simulink model "VirtualADAPT"  (Simulink 8.9) (continued)
== system VirtualADAPT/VirtualADAPTv1/PowerDistribution/SensorIT281 ==
  TrueValue : Inport
  MSe : Subsystem  mask{FP=Nominal, FT=0, FM=0}
      mask prompts: FP "Fault Profile:"; FT "Fault Injection Time:"; FM "Fault Magnitude/Slope/Bias/StuckAtValue"
      mask TypeOptions FP: Nominal | Abrupt | Incipient | Bias | StuckAt
      mask Display: image(imread('MSe.png','BackgroundColor',[1 1 1]))
  R : Subsystem  mask{R=1, FP=Nominal, FT=0, FM=0}
      mask prompts: R "Value of R:"; FP "Fault Profile:"; FT "Fault Injection Time:"; FM "Fault Magnitude/Slope"
      mask TypeOptions FP: Nominal | Abrupt | Incipient
      mask Display: image(imread('R.png','BackgroundColor',[1 1 1]))
  TrueValueMod : Subsystem
      mask Display: image(imread('fx.png','BackgroundColor',[1 1 1]))
  Value : Subsystem
      mask Display: image(imread('0.png','BackgroundColor',[1 1 1]))
  SensedValue : Outport
  wires:
  Value/1(y(1)) -> R/1(In)
  R/1(Out) -> Value/1(u(1))
  MSe/1(E) -> Value/2(u(2))
  Value/2(y(2)) -> MSe/1(F)
  TrueValueMod/1(y(1)) -> MSe/2(Se)
  TrueValue/1 -> TrueValueMod/1(u(1))
  Value/3(O) -> SensedValue/1

== system VirtualADAPT/VirtualADAPTv1/PowerDistribution/SensorST165 ==
  TrueValue : Inport
  MSe : Subsystem  mask{FP=Nominal, FT=0, FM=0}
      mask prompts: FP "Fault Profile:"; FT "Fault Injection Time:"; FM "Fault Magnitude/Slope/Bias/StuckAtValue"
      mask TypeOptions FP: Nominal | Abrupt | Incipient | Bias | StuckAt
      mask Display: image(imread('MSe.png','BackgroundColor',[1 1 1]))
  R : Subsystem  mask{R=1, FP=Nominal, FT=0, FM=0}
      mask prompts: R "Value of R:"; FP "Fault Profile:"; FT "Fault Injection Time:"; FM "Fault Magnitude/Slope"
      mask TypeOptions FP: Nominal | Abrupt | Incipient
      mask Display: image(imread('R.png','BackgroundColor',[1 1 1]))
  TrueValueMod : Subsystem
      mask Display: image(imread('fx.png','BackgroundColor',[1 1 1]))
  Value : Subsystem
      mask Display: image(imread('0.png','BackgroundColor',[1 1 1]))
  SensedValue : Outport
  wires:
  Value/1(y(1)) -> R/1(In)
  R/1(Out) -> Value/1(u(1))
  MSe/1(E) -> Value/2(u(2))
  Value/2(y(2)) -> MSe/1(F)
  TrueValueMod/1(y(1)) -> MSe/2(Se)
  TrueValue/1 -> TrueValueMod/1(u(1))
  Value/3(O) -> SensedValue/1

== system VirtualADAPT/VirtualADAPTv1/PowerDistribution/SensorST265 ==
  TrueValue : Inport
  MSe : Subsystem  mask{FP=Nominal, FT=0, FM=0}
      mask prompts: FP "Fault Profile:"; FT "Fault Injection Time:"; FM "Fault Magnitude/Slope/Bias/StuckAtValue"
      mask TypeOptions FP: Nominal | Abrupt | Incipient | Bias | StuckAt
      mask Display: image(imread('MSe.png','BackgroundColor',[1 1 1]))
  R : Subsystem  mask{R=1, FP=Nominal, FT=0, FM=0}
      mask prompts: R "Value of R:"; FP "Fault Profile:"; FT "Fault Injection Time:"; FM "Fault Magnitude/Slope"
      mask TypeOptions FP: Nominal | Abrupt | Incipient
      mask Display: image(imread('R.png','BackgroundColor',[1 1 1]))
  TrueValueMod : Subsystem
      mask Display: image(imread('fx.png','BackgroundColor',[1 1 1]))
  Value : Subsystem
      mask Display: image(imread('0.png','BackgroundColor',[1 1 1]))
  SensedValue : Outport
  wires:
  Value/1(y(1)) -> R/1(In)
  R/1(Out) -> Value/1(u(1))
  MSe/1(E) -> Value/2(u(2))
  Value/2(y(2)) -> MSe/1(F)
  TrueValueMod/1(y(1)) -> MSe/2(Se)
  TrueValue/1 -> TrueValueMod/1(u(1))
  Value/3(O) -> SensedValue/1

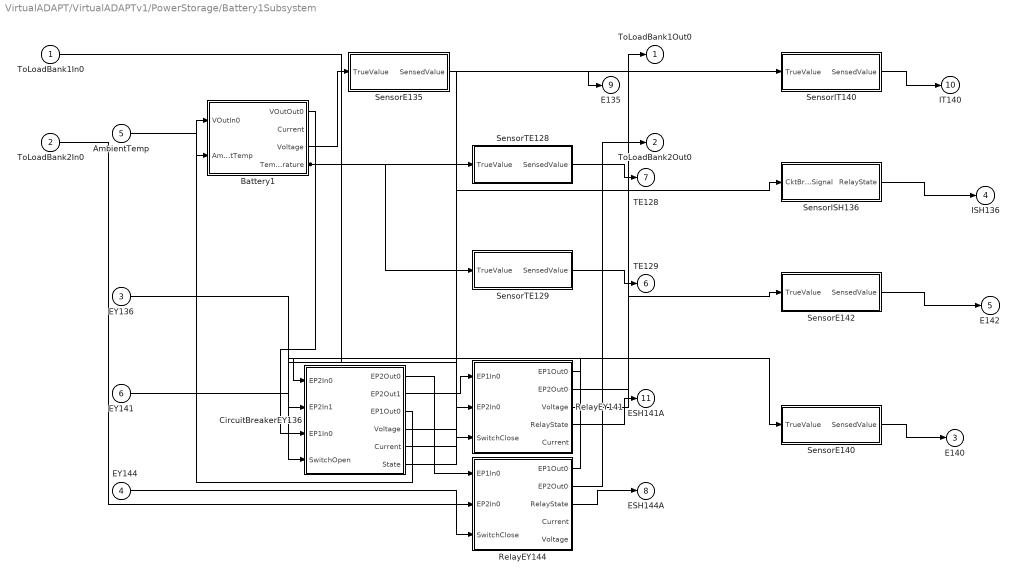
== system VirtualADAPT/VirtualADAPTv1/PowerStorage/Battery1Subsystem ==
  ToLoadBank1In0 : Inport
  ToLoadBank2In0 : Inport  Port=2
  EY136 : Inport  Port=3
  EY144 : Inport  Port=4
  AmbientTemp : Inport  Port=5
  EY141 : Inport  Port=6
  Battery1 : Subsystem
  CircuitBreakerEY136 : Subsystem
  RelayEY141 : Subsystem
  RelayEY144 : Subsystem
  SensorE135 : Subsystem
  SensorE140 : Subsystem
  SensorE142 : Subsystem
  SensorISH136 : Subsystem
  SensorIT140 : Subsystem
  SensorTE128 : Subsystem
  SensorTE129 : Subsystem
  ToLoadBank1Out0 : Outport
  ToLoadBank2Out0 : Outport  Port=2
  E140 : Outport  Port=3
  ISH136 : Outport  Port=4
  E142 : Outport  Port=5
  TE129 : Outport  Port=6
  TE128 : Outport  Port=7
  ESH144A : Outport  Port=8
  E135 : Outport  Port=9
  IT140 : Outport  Port=10
  ESH141A : Outport  Port=11
  wires:
  CircuitBreakerEY136/1(EP2Out0) -> RelayEY144/1(EP1In0)
  RelayEY144/1(EP1Out0) -> CircuitBreakerEY136/1(EP2In0)
  CircuitBreakerEY136/2(EP2Out1) -> RelayEY141/1(EP1In0)
  RelayEY141/1(EP1Out0) -> CircuitBreakerEY136/2(EP2In1)
  RelayEY144/2(EP2Out0) -> ToLoadBank2Out0/1
  ToLoadBank2In0/1 -> RelayEY144/2(EP2In0)
  RelayEY141/2(EP2Out0) -> ToLoadBank1Out0/1
  ToLoadBank1In0/1 -> RelayEY141/2(EP2In0)
  Battery1/1(VOutOut0) -> CircuitBreakerEY136/3(EP1In0)
  CircuitBreakerEY136/3(EP1Out0) -> Battery1/1(VOutIn0)
  Battery1/3(Voltage) -> SensorE135/1(TrueValue)
  CircuitBreakerEY136/4(Voltage) -> SensorE140/1(TrueValue)
  SensorE140/1(SensedValue) -> E140/1
  CircuitBreakerEY136/5(Current) -> SensorIT140/1(TrueValue)
  SensorISH136/1(RelayState) -> ISH136/1
  CircuitBreakerEY136/6(State) -> SensorISH136/1(CktBrkOpenSignal)
  EY136/1 -> CircuitBreakerEY136/4(SwitchOpen)
  SensorE142/1(SensedValue) -> E142/1
  RelayEY141/3(Voltage) -> SensorE142/1(TrueValue)
  SensorTE129/1(SensedValue) -> TE129/1
  SensorTE128/1(SensedValue) -> TE128/1
  RelayEY144/3(RelayState) -> ESH144A/1
  RelayEY141/4(RelayState) -> ESH141A/1
  SensorIT140/1(SensedValue) -> IT140/1
  SensorE135/1(SensedValue) -> E135/1
  EY141/1 -> RelayEY141/3(SwitchClose)
  EY144/1 -> RelayEY144/3(SwitchClose)
  Battery1/4(Temperature) -> SensorTE128/1(TrueValue), SensorTE129/1(TrueValue)
  AmbientTemp/1 -> Battery1/2(AmbientTemp)

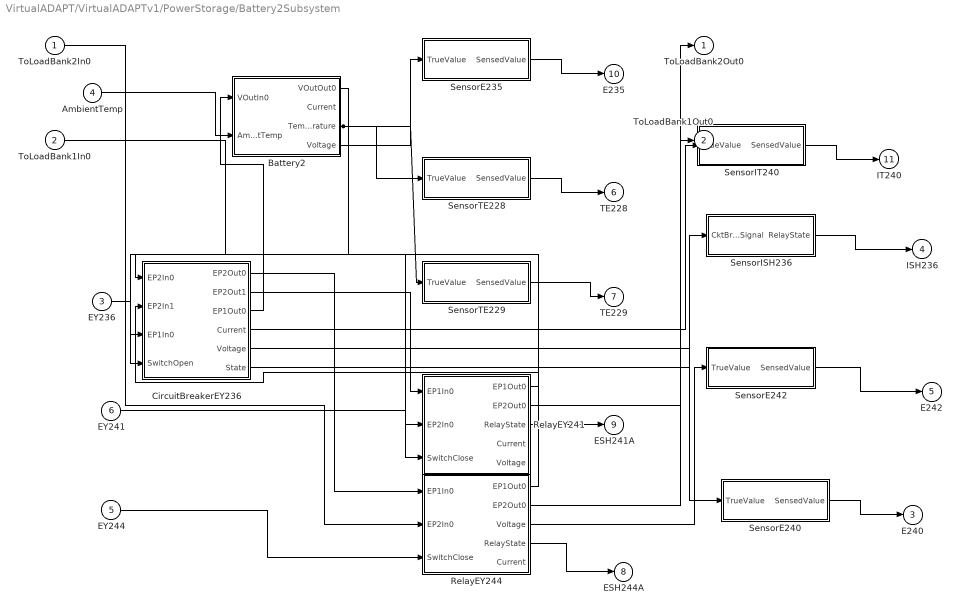
== system VirtualADAPT/VirtualADAPTv1/PowerStorage/Battery2Subsystem ==
  ToLoadBank2In0 : Inport
  ToLoadBank1In0 : Inport  Port=2
  EY236 : Inport  Port=3
  AmbientTemp : Inport  Port=4
  EY244 : Inport  Port=5
  EY241 : Inport  Port=6
  Battery2 : Subsystem
  CircuitBreakerEY236 : Subsystem
  RelayEY241 : Subsystem
  RelayEY244 : Subsystem
  SensorE235 : Subsystem
  SensorE240 : Subsystem
  SensorE242 : Subsystem
  SensorISH236 : Subsystem
  SensorIT240 : Subsystem
  SensorTE228 : Subsystem
  SensorTE229 : Subsystem
  ToLoadBank2Out0 : Outport
  ToLoadBank1Out0 : Outport  Port=2
  E240 : Outport  Port=3
  ISH236 : Outport  Port=4
  E242 : Outport  Port=5
  TE228 : Outport  Port=6
  TE229 : Outport  Port=7
  ESH244A : Outport  Port=8
  ESH241A : Outport  Port=9
  E235 : Outport  Port=10
  IT240 : Outport  Port=11
  wires:
  CircuitBreakerEY236/1(EP2Out0) -> RelayEY244/1(EP1In0)
  RelayEY244/1(EP1Out0) -> CircuitBreakerEY236/1(EP2In0)
  CircuitBreakerEY236/2(EP2Out1) -> RelayEY241/1(EP1In0)
  RelayEY241/1(EP1Out0) -> CircuitBreakerEY236/2(EP2In1)
  RelayEY244/2(EP2Out0) -> ToLoadBank2Out0/1
  ToLoadBank2In0/1 -> RelayEY244/2(EP2In0)
  RelayEY241/2(EP2Out0) -> ToLoadBank1Out0/1
  ToLoadBank1In0/1 -> RelayEY241/2(EP2In0)
  Battery2/1(VOutOut0) -> CircuitBreakerEY236/3(EP1In0)
  CircuitBreakerEY236/3(EP1Out0) -> Battery2/1(VOutIn0)
  Battery2/3(Temperature) -> SensorTE229/1(TrueValue), SensorTE228/1(TrueValue)
  RelayEY244/3(Voltage) -> SensorE242/1(TrueValue)
  SensorE240/1(SensedValue) -> E240/1
  CircuitBreakerEY236/6(State) -> SensorISH236/1(CktBrkOpenSignal)
  SensorISH236/1(RelayState) -> ISH236/1
  EY236/1 -> CircuitBreakerEY236/4(SwitchOpen)
  SensorE242/1(SensedValue) -> E242/1
  SensorTE229/1(SensedValue) -> TE229/1
  SensorTE228/1(SensedValue) -> TE228/1
  RelayEY244/4(RelayState) -> ESH244A/1
  RelayEY241/3(RelayState) -> ESH241A/1
  SensorIT240/1(SensedValue) -> IT240/1
  SensorE235/1(SensedValue) -> E235/1
  EY244/1 -> RelayEY244/3(SwitchClose)
  EY241/1 -> RelayEY241/3(SwitchClose)
  Battery2/4(Voltage) -> SensorE235/1(TrueValue)
  AmbientTemp/1 -> Battery2/2(AmbientTemp)
  CircuitBreakerEY236/4(Current) -> SensorIT240/1(TrueValue)
  CircuitBreakerEY236/5(Voltage) -> SensorE240/1(TrueValue)

== system VirtualADAPT/VirtualADAPTv1/PowerStorage/RelayEY160 ==
  EP1In0 : Inport
  EP1In1 : Inport  Port=2
  EP2In0 : Inport  Port=3
  EP2In1 : Inport  Port=4
  SwitchClose : Inport  Port=5
  ParallelConn : Subsystem
      mask Display: image(imread('0.png','BackgroundColor',[1 1 1]))
  Relay : Subsystem
      mask Display: image(imread('1.png','BackgroundColor',[1 1 1]))
  Sensor : Subsystem
  EP1Out0 : Outport
  EP1Out1 : Outport  Port=2
  EP2Out0 : Outport  Port=3
  EP2Out1 : Outport  Port=4
  Current : Outport  Port=5
  Voltage : Outport  Port=6
  RelayState : Outport  Port=7
  wires:
  Relay/1(y(1)) -> ParallelConn/1(u(1))
  ParallelConn/1(y(1)) -> Relay/1(u(1))
  EP2In0/1 -> ParallelConn/2(u(2))
  ParallelConn/2(y(2)) -> EP2Out0/1
  EP2In1/1 -> ParallelConn/3(u(3))
  ParallelConn/3(y(3)) -> EP2Out1/1
  EP1In0/1 -> Relay/2(u(2))
  Relay/2(y(2)) -> EP1Out0/1
  EP1In1/1 -> Relay/3(u(3))
  Relay/3(y(3)) -> EP1Out1/1
  SwitchClose/1 -> Relay/4(u(4)), ParallelConn/4(u(4))
  Relay/4(O) -> Current/1
  ParallelConn/4(O) -> Voltage/1
  Sensor/1(SensedValue) -> RelayState/1
  Relay/5(State) -> Sensor/1(TrueValue)

== system VirtualADAPT/VirtualADAPTv1/PowerStorage/RelayEY260 ==
  EP1In0 : Inport
  EP1In1 : Inport  Port=2
  EP2In0 : Inport  Port=3
  EP2In1 : Inport  Port=4
  SwitchClose : Inport  Port=5
  ParallelConn : Subsystem
      mask Display: image(imread('0.png','BackgroundColor',[1 1 1]))
  Relay : Subsystem
      mask Display: image(imread('1.png','BackgroundColor',[1 1 1]))
  Sensor : Subsystem
  EP1Out0 : Outport
  EP1Out1 : Outport  Port=2
  EP2Out0 : Outport  Port=3
  EP2Out1 : Outport  Port=4
  Current : Outport  Port=5
  Voltage : Outport  Port=6
  RelayState : Outport  Port=7
  wires:
  Relay/1(y(1)) -> ParallelConn/1(u(1))
  ParallelConn/1(y(1)) -> Relay/1(u(1))
  EP2In0/1 -> ParallelConn/2(u(2))
  ParallelConn/2(y(2)) -> EP2Out0/1
  EP2In1/1 -> ParallelConn/3(u(3))
  ParallelConn/3(y(3)) -> EP2Out1/1
  EP1In0/1 -> Relay/2(u(2))
  Relay/2(y(2)) -> EP1Out0/1
  EP1In1/1 -> Relay/3(u(3))
  Relay/3(y(3)) -> EP1Out1/1
  SwitchClose/1 -> Relay/4(u(4)), ParallelConn/4(u(4))
  Relay/4(O) -> Current/1
  ParallelConn/4(O) -> Voltage/1
  Sensor/1(SensedValue) -> RelayState/1
  Relay/5(State) -> Sensor/1(TrueValue)

== system VirtualADAPT/VirtualADAPTv1/PowerStorage/SensorE161 ==
  TrueValue : Inport
  MSe : Subsystem  mask{FP=Nominal, FT=0, FM=0}
      mask prompts: FP "Fault Profile:"; FT "Fault Injection Time:"; FM "Fault Magnitude/Slope/Bias/StuckAtValue"
      mask TypeOptions FP: Nominal | Abrupt | Incipient | Bias | StuckAt
      mask Display: image(imread('MSe.png','BackgroundColor',[1 1 1]))
  R : Subsystem  mask{R=1, FP=Nominal, FT=0, FM=0}
      mask prompts: R "Value of R:"; FP "Fault Profile:"; FT "Fault Injection Time:"; FM "Fault Magnitude/Slope"
      mask TypeOptions FP: Nominal | Abrupt | Incipient
      mask Display: image(imread('R.png','BackgroundColor',[1 1 1]))
  TrueValueMod : Subsystem
      mask Display: image(imread('fx.png','BackgroundColor',[1 1 1]))
  Value : Subsystem
      mask Display: image(imread('0.png','BackgroundColor',[1 1 1]))
  SensedValue : Outport
  wires:
  MSe/1(E) -> Value/1(u(1))
  Value/1(y(1)) -> MSe/1(F)
  Value/2(y(2)) -> R/1(In)
  R/1(Out) -> Value/2(u(2))
  TrueValueMod/1(y(1)) -> MSe/2(Se)
  TrueValue/1 -> TrueValueMod/1(u(1))
  Value/3(O) -> SensedValue/1

== system VirtualADAPT/VirtualADAPTv1/PowerStorage/SensorE261 ==
  TrueValue : Inport
  MSe : Subsystem  mask{FP=Nominal, FT=0, FM=0}
      mask prompts: FP "Fault Profile:"; FT "Fault Injection Time:"; FM "Fault Magnitude/Slope/Bias/StuckAtValue"
      mask TypeOptions FP: Nominal | Abrupt | Incipient | Bias | StuckAt
      mask Display: image(imread('MSe.png','BackgroundColor',[1 1 1]))
  R : Subsystem  mask{R=1, FP=Nominal, FT=0, FM=0}
      mask prompts: R "Value of R:"; FP "Fault Profile:"; FT "Fault Injection Time:"; FM "Fault Magnitude/Slope"
      mask TypeOptions FP: Nominal | Abrupt | Incipient
      mask Display: image(imread('R.png','BackgroundColor',[1 1 1]))
  TrueValueMod : Subsystem
      mask Display: image(imread('fx.png','BackgroundColor',[1 1 1]))
  Value : Subsystem
      mask Display: image(imread('0.png','BackgroundColor',[1 1 1]))
  SensedValue : Outport
  wires:
  MSe/1(E) -> Value/1(u(1))
  Value/1(y(1)) -> MSe/1(F)
  Value/2(y(2)) -> R/1(In)
  R/1(Out) -> Value/2(u(2))
  TrueValueMod/1(y(1)) -> MSe/2(Se)
  TrueValue/1 -> TrueValueMod/1(u(1))
  Value/3(O) -> SensedValue/1

== system VirtualADAPT/VirtualADAPTv1/PowerStorage/SensorIT161 ==
  TrueValue : Inport
  MSe : Subsystem  mask{FP=Nominal, FT=0, FM=0}
      mask prompts: FP "Fault Profile:"; FT "Fault Injection Time:"; FM "Fault Magnitude/Slope/Bias/StuckAtValue"
      mask TypeOptions FP: Nominal | Abrupt | Incipient | Bias | StuckAt
      mask Display: image(imread('MSe.png','BackgroundColor',[1 1 1]))
  R : Subsystem  mask{R=1, FP=Nominal, FT=0, FM=0}
      mask prompts: R "Value of R:"; FP "Fault Profile:"; FT "Fault Injection Time:"; FM "Fault Magnitude/Slope"
      mask TypeOptions FP: Nominal | Abrupt | Incipient
      mask Display: image(imread('R.png','BackgroundColor',[1 1 1]))
  TrueValueMod : Subsystem
      mask Display: image(imread('fx.png','BackgroundColor',[1 1 1]))
  Value : Subsystem
      mask Display: image(imread('0.png','BackgroundColor',[1 1 1]))
  SensedValue : Outport
  wires:
  MSe/1(E) -> Value/1(u(1))
  Value/1(y(1)) -> MSe/1(F)
  Value/2(y(2)) -> R/1(In)
  R/1(Out) -> Value/2(u(2))
  TrueValueMod/1(y(1)) -> MSe/2(Se)
  TrueValue/1 -> TrueValueMod/1(u(1))
  Value/3(O) -> SensedValue/1

== system VirtualADAPT/VirtualADAPTv1/PowerStorage/SensorIT261 ==
  TrueValue : Inport
  MSe : Subsystem  mask{FP=Nominal, FT=0, FM=0}
      mask prompts: FP "Fault Profile:"; FT "Fault Injection Time:"; FM "Fault Magnitude/Slope/Bias/StuckAtValue"
      mask TypeOptions FP: Nominal | Abrupt | Incipient | Bias | StuckAt
      mask Display: image(imread('MSe.png','BackgroundColor',[1 1 1]))
  R : Subsystem  mask{R=1, FP=Nominal, FT=0, FM=0}
      mask prompts: R "Value of R:"; FP "Fault Profile:"; FT "Fault Injection Time:"; FM "Fault Magnitude/Slope"
      mask TypeOptions FP: Nominal | Abrupt | Incipient
      mask Display: image(imread('R.png','BackgroundColor',[1 1 1]))
  TrueValueMod : Subsystem
      mask Display: image(imread('fx.png','BackgroundColor',[1 1 1]))
  Value : Subsystem
      mask Display: image(imread('0.png','BackgroundColor',[1 1 1]))
  SensedValue : Outport
  wires:
  MSe/1(E) -> Value/1(u(1))
  Value/1(y(1)) -> MSe/1(F)
  Value/2(y(2)) -> R/1(In)
  R/1(Out) -> Value/2(u(2))
  TrueValueMod/1(y(1)) -> MSe/2(Se)
  TrueValue/1 -> TrueValueMod/1(u(1))
  Value/3(O) -> SensedValue/1

== system VirtualADAPT/VirtualADAPTv1/SensorTE133/MSe ==
  F : Inport
  Se : Inport  Port=2
  Bias : Step  Time=FT  After=FM  SampleTime=0
  Fault Inject : Subsystem
  Fault Profile : Constant  Value=FP
  Mult2 : Product  SaturateOnIntegerOverflow=on  RndMeth=Zero  InputSameDT=on  OutDataTypeStr=Inherit: Same as first input
  Multiply : Product  SaturateOnIntegerOverflow=on  RndMeth=Zero  InputSameDT=on  OutDataTypeStr=Inherit: Same as first input
  Ramp : simulink/Sources/Ramp  slope=FM  start=FT  X0=0
  Step : Step  Time=FT  Before=1  After=(1 + FM)  SampleTime=0
  StuckAt : Step  Time=FT  Before=1  After=0  SampleTime=0
  StuckAt2 : Step  Time=FT  After=FM  SampleTime=0
  Sum : Sum  SaturateOnIntegerOverflow=on  InputSameDT=on  OutDataTypeStr=Inherit: Same as first input
  Sum2 : Sum  SaturateOnIntegerOverflow=on  InputSameDT=on  OutDataTypeStr=Inherit: Same as first input
  Sum3 : Sum  SaturateOnIntegerOverflow=on  InputSameDT=on  OutDataTypeStr=Inherit: Same as first input
  E : Outport
  wires:
  Fault Inject/1(Out1) -> E/1
  Se/1 -> Fault Inject/1(Nominal), Multiply/1, Sum/1, Sum2/1, Mult2/1
  Fault Profile/1 -> Fault Inject/2(Fault Profile)
  Step/1 -> Multiply/2
  Ramp/1 -> Sum/2
  Multiply/1 -> Fault Inject/3(Step)
  Sum/1 -> Fault Inject/4(Ramp)
  Bias/1 -> Sum2/2
  Sum2/1 -> Fault Inject/5(Bias)
  StuckAt/1 -> Mult2/2
  Mult2/1 -> Sum3/1
  StuckAt2/1 -> Sum3/2
  Sum3/1 -> Fault Inject/6(stuckAt)

== system VirtualADAPT/VirtualADAPTv1/SensorTE133/R ==
  In : Inport
  Bond : Constant
  Computing R : Subsystem
  Fault Profile : Constant  Value=FP
  JuncDB : Constant  Value=2
  Junction Type : Constant  Value=0
  Ramp : simulink/Sources/Ramp  slope=FM  start=FT  X0=R
  Resistor Value : Constant  Value=R
  Step : Step  Time=FT  Before=R  After=(1 + FM)*R  SampleTime=0
  Out : Outport
  R : Outport  Port=2
  wires:
  Computing R/1(Out1) -> Out/1
  In/1 -> Computing R/1(In1)
  Resistor Value/1 -> Computing R/2(RNominal)
  Fault Profile/1 -> Computing R/3(Fault Profile)
  Step/1 -> Computing R/4(Step)
  Ramp/1 -> Computing R/5(Ramp)
  JuncDB/1 -> Computing R/6(JuncDB)
  Bond/1 -> Computing R/7(Bond)
  Junction Type/1 -> Computing R/8(Junction Type)
  Computing R/2(Out2) -> R/1

== system VirtualADAPT/VirtualADAPTv1/SensorTE133/TempBias ==
  Constant : Constant  Value=Constant
  Fault Inject : Subsystem
  Fault Profile : Constant  Value=FP
  Ramp : simulink/Sources/Ramp  slope=FM  start=FT  X0=Constant
  Step : Step  Time=FT  Before=Constant  After=(1 + FM)*Constant  SampleTime=0
  C : Outport
  wires:
  Fault Inject/1(Out1) -> C/1
  Constant/1 -> Fault Inject/1(Nominal)
  Fault Profile/1 -> Fault Inject/2(Fault Profile)
  Step/1 -> Fault Inject/3(Step)
  Ramp/1 -> Fault Inject/4(Ramp)

== system VirtualADAPT/VirtualADAPTv1/SensorTE133/TrueValueMod ==
  u(1) : Inport
  u(2) : Inport  Port=2
  Function : Fcn  Expr=(u(1)+u(2))*9/5+32
  Mux : Mux  Inputs=2
  y(1) : Outport
  wires:
  u(1)/1 -> Mux/1
  u(2)/1 -> Mux/2
  Mux/1 -> Function/1
  Function/1 -> y(1)/1

== system VirtualADAPT/VirtualADAPTv1/SensorTE133/Value ==
  u(1) : Inport
  u(2) : Inport  Port=2
  Adder : Sum  Inputs=-  SaturateOnIntegerOverflow=on  InputSameDT=on  OutDataTypeStr=Inherit: Same as first input
  Gain : Gain  Gain=-1  SaturateOnIntegerOverflow=on  OutDataTypeStr=Inherit: Same as input  ParamDataTypeStr=Inherit: Same as input
  y(1) : Outport
  y(2) : Outport  Port=2
  O : Outport  Port=3
  wires:
  Adder/1 -> Gain/1
  u(2)/1 -> y(1)/1, O/1
  u(1)/1 -> Adder/1
  Gain/1 -> y(2)/1

== system VirtualADAPT/VirtualADAPTv1/AmbientTemp/AmbientTemp/Fault Inject ==
  Nominal : Inport
  Fault Profile : Inport  Port=2
  Step : Inport  Port=3
  Ramp : Inport  Port=4
  Bias : Inport  Port=5
  stuckAt : Inport  Port=6
  Switch1 : MultiPortSwitch  Inputs=5  SaturateOnIntegerOverflow=on  InputSameDT=on
  Out1 : Outport
  wires:
  Nominal/1 -> Switch1/2
  Fault Profile/1 -> Switch1/1
  Step/1 -> Switch1/3
  Ramp/1 -> Switch1/4
  Bias/1 -> Switch1/5
  stuckAt/1 -> Switch1/6
  Switch1/1 -> Out1/1

== system VirtualADAPT/VirtualADAPTv1/PowerDistribution/ACLoadBank1/L1A ==
  VInIn0 : Inport
  AmbientTemp : Inport  Port=2
  LGT6 : Subsystem
  SensorLT500 : Subsystem
  SensorTE500 : Subsystem
  SensorTE501 : Subsystem
  SensorTE502 : Subsystem
  VInOut0 : Outport
  TE502 : Outport  Port=2
  TE501 : Outport  Port=3
  TE500 : Outport  Port=4
  LT500 : Outport  Port=5
  wires:
  VInIn0/1 -> LGT6/1(VInIn0)
  LGT6/1(VInOut0) -> VInOut0/1
  LGT6/4(Temperature3) -> SensorTE502/1(TrueValue)
  LGT6/6(Temperature2) -> SensorTE501/1(TrueValue)
  SensorTE502/1(SensedValue) -> TE502/1
  SensorTE501/1(SensedValue) -> TE501/1
  SensorTE500/1(SensedValue) -> TE500/1
  SensorLT500/1(Light) -> LT500/1
  LGT6/3(Temperature1) -> SensorTE500/1(TrueValue)
  LGT6/2(Power) -> SensorLT500/1(Power)
  AmbientTemp/1 -> LGT6/2(AmbientTemp)

== system VirtualADAPT/VirtualADAPTv1/PowerDistribution/ACLoadBank1/L1B ==
  VInIn0 : Inport
  FAN1 : Subsystem
  SensorST515 : Subsystem
  VInOut0 : Outport
  ST515 : Outport  Port=2
  Current : Outport  Port=3
  wires:
  VInIn0/1 -> FAN1/1(InputIn0)
  FAN1/1(InputOut0) -> VInOut0/1
  FAN1/3(Current) -> Current/1
  FAN1/2(RotationalVelocity) -> SensorST515/1(TrueValue)
  SensorST515/1(SensedValue) -> ST515/1

== system VirtualADAPT/VirtualADAPTv1/PowerDistribution/ACLoadBank1/L1C ==
  EnergyPortIn0 : Inport
  FAN3 : Subsystem
  EnergyPortOut0 : Outport
  wires:
  EnergyPortIn0/1 -> FAN3/1(EPIn0)
  FAN3/1(EPOut0) -> EnergyPortOut0/1

== system VirtualADAPT/VirtualADAPTv1/PowerDistribution/ACLoadBank1/L1D ==
  EnergyPortIn0 : Inport
  LGT8 : Subsystem
  EnergyPortOut0 : Outport
  wires:
  EnergyPortIn0/1 -> LGT8/1(EPIn0)
  LGT8/1(EPOut0) -> EnergyPortOut0/1

== system VirtualADAPT/VirtualADAPTv1/PowerDistribution/ACLoadBank1/L1E ==
  VInIn0 : Inport
  PMP2 : Subsystem
  SensorFT525 : Subsystem
  VInOut0 : Outport
  FT525 : Outport  Port=2
  wires:
  VInIn0/1 -> PMP2/1(InputIn0)
  PMP2/1(InputOut0) -> VInOut0/1
  PMP2/3(Flow) -> SensorFT525/1(TrueValue)
  SensorFT525/1(SensedValue) -> FT525/1

== system VirtualADAPT/VirtualADAPTv1/PowerDistribution/ACLoadBank1/L1F ==
  InputIn0 : Inport
  AmbientTemp : Inport  Port=2
  LGT4 : Subsystem
  SensorTE511 : Subsystem
  InputOut0 : Outport
  TE511 : Outport  Port=2
  Current : Outport  Port=3
  wires:
  InputIn0/1 -> LGT4/1(EPIn0)
  LGT4/1(EPOut0) -> InputOut0/1
  AmbientTemp/1 -> LGT4/2(AmbientTemp)
  LGT4/3(Current) -> Current/1
  LGT4/4(Temperature) -> SensorTE511/1(TrueValue)
  SensorTE511/1(SensedValue) -> TE511/1

== system VirtualADAPT/VirtualADAPTv1/PowerDistribution/ACLoadBank1/RelayEY170 ==
  EP2In0 : Inport
  EP1In0 : Inport  Port=2
  SwitchClose : Inport  Port=3
  ParallelConn : Subsystem
      mask Display: image(imread('0.png','BackgroundColor',[1 1 1]))
  Relay : Subsystem
      mask Display: image(imread('1.png','BackgroundColor',[1 1 1]))
  Sensor : Subsystem
  EP2Out0 : Outport
  EP1Out0 : Outport  Port=2
  RelayState : Outport  Port=3
  Current : Outport  Port=4
  Voltage : Outport  Port=5
  wires:
  Relay/1(y(1)) -> ParallelConn/1(u(1))
  ParallelConn/1(y(1)) -> Relay/1(u(1))
  EP2In0/1 -> ParallelConn/2(u(2))
  ParallelConn/2(y(2)) -> EP2Out0/1
  EP1In0/1 -> Relay/2(u(2))
  Relay/2(y(2)) -> EP1Out0/1
  SwitchClose/1 -> Relay/3(u(3)), ParallelConn/3(u(3))
  Relay/3(O) -> Current/1
  ParallelConn/3(O) -> Voltage/1
  Sensor/1(SensedValue) -> RelayState/1
  Relay/4(State) -> Sensor/1(TrueValue)

== system VirtualADAPT/VirtualADAPTv1/PowerDistribution/ACLoadBank1/RelayEY171 ==
  EP2In0 : Inport
  EP1In0 : Inport  Port=2
  SwitchClose : Inport  Port=3
  ParallelConn : Subsystem
      mask Display: image(imread('0.png','BackgroundColor',[1 1 1]))
  Relay : Subsystem
      mask Display: image(imread('1.png','BackgroundColor',[1 1 1]))
  Sensor : Subsystem
  EP2Out0 : Outport
  EP1Out0 : Outport  Port=2
  RelayState : Outport  Port=3
  Current : Outport  Port=4
  Voltage : Outport  Port=5
  wires:
  Relay/1(y(1)) -> ParallelConn/1(u(1))
  ParallelConn/1(y(1)) -> Relay/1(u(1))
  EP2In0/1 -> ParallelConn/2(u(2))
  ParallelConn/2(y(2)) -> EP2Out0/1
  EP1In0/1 -> Relay/2(u(2))
  Relay/2(y(2)) -> EP1Out0/1
  SwitchClose/1 -> Relay/3(u(3)), ParallelConn/3(u(3))
  Relay/3(O) -> Current/1
  ParallelConn/3(O) -> Voltage/1
  Sensor/1(SensedValue) -> RelayState/1
  Relay/4(State) -> Sensor/1(TrueValue)

== system VirtualADAPT/VirtualADAPTv1/PowerDistribution/ACLoadBank1/RelayEY172 ==
  EP2In0 : Inport
  EP1In0 : Inport  Port=2
  SwitchClose : Inport  Port=3
  ParallelConn : Subsystem
      mask Display: image(imread('0.png','BackgroundColor',[1 1 1]))
  Relay : Subsystem
      mask Display: image(imread('1.png','BackgroundColor',[1 1 1]))
  Sensor : Subsystem
  EP2Out0 : Outport
  EP1Out0 : Outport  Port=2
  RelayState : Outport  Port=3
  Current : Outport  Port=4
  Voltage : Outport  Port=5
  wires:
  Relay/1(y(1)) -> ParallelConn/1(u(1))
  ParallelConn/1(y(1)) -> Relay/1(u(1))
  EP2In0/1 -> ParallelConn/2(u(2))
  ParallelConn/2(y(2)) -> EP2Out0/1
  EP1In0/1 -> Relay/2(u(2))
  Relay/2(y(2)) -> EP1Out0/1
  SwitchClose/1 -> Relay/3(u(3)), ParallelConn/3(u(3))
  Relay/3(O) -> Current/1
  ParallelConn/3(O) -> Voltage/1
  Sensor/1(SensedValue) -> RelayState/1
  Relay/4(State) -> Sensor/1(TrueValue)

== system VirtualADAPT/VirtualADAPTv1/PowerDistribution/ACLoadBank1/RelayEY173 ==
  EP2In0 : Inport
  EP1In0 : Inport  Port=2
  SwitchClose : Inport  Port=3
  ParallelConn : Subsystem
      mask Display: image(imread('0.png','BackgroundColor',[1 1 1]))
  Relay : Subsystem
      mask Display: image(imread('1.png','BackgroundColor',[1 1 1]))
  Sensor : Subsystem
  EP2Out0 : Outport
  EP1Out0 : Outport  Port=2
  RelayState : Outport  Port=3
  Current : Outport  Port=4
  Voltage : Outport  Port=5
  wires:
  Relay/1(y(1)) -> ParallelConn/1(u(1))
  ParallelConn/1(y(1)) -> Relay/1(u(1))
  EP2In0/1 -> ParallelConn/2(u(2))
  ParallelConn/2(y(2)) -> EP2Out0/1
  EP1In0/1 -> Relay/2(u(2))
  Relay/2(y(2)) -> EP1Out0/1
  SwitchClose/1 -> Relay/3(u(3)), ParallelConn/3(u(3))
  Relay/3(O) -> Current/1
  ParallelConn/3(O) -> Voltage/1
  Sensor/1(SensedValue) -> RelayState/1
  Relay/4(State) -> Sensor/1(TrueValue)
